simulink model "cddDcmApp"  (R2022b) (continued)
== system cddDcmApp/cddDcmApp_20ms_Runnable_sys == (continued)
  VgaIOCtrlService_FreezeMotorCurrentPosition : FunctionCaller  FunctionPrototype=ERR = VgaIOCtrlService_FreezeMotorCurrentPosition(Channel)  InputArgumentSpecifications=VGAChlType(0)  OutputArgumentSpecifications=P_Std_ReturnType
  VgaIOCtrlService_MotorReturnToECU : FunctionCaller  FunctionPrototype=ERR = VgaIOCtrlService_MotorReturnToECU(Channel)  InputArgumentSpecifications=VGAChlType(0)  OutputArgumentSpecifications=P_Std_ReturnType
  VgaIOCtrlService_MotorShortTermAdj : FunctionCaller  FunctionPrototype=ERR = VgaIOCtrlService_MotorShortTermAdj(Channel,TempValue)  InputArgumentSpecifications=VGAChlType(0), uint16(1)  OutputArgumentSpecifications=P_Std_ReturnType
  VgaParaService_GetAllMotorCaliStatus : FunctionCaller  FunctionPrototype=[Status,ERR] = VgaParaService_GetAllMotorCaliStatus()  OutputArgumentSpecifications=uint8(1), P_Std_ReturnType
  VgaParaService_GetCailRoutineStatus : FunctionCaller  FunctionPrototype=[Status,ERR] = VgaParaService_GetCailRoutineStatus()  OutputArgumentSpecifications=MtrRoutStaEnum(0), P_Std_ReturnType
  VgaParaService_GetFreshRecErrorStatus : FunctionCaller  FunctionPrototype=[Status,ERR] = VgaParaService_GetFreshRecErrorStatus()  OutputArgumentSpecifications=uint8(1), P_Std_ReturnType
  VgaParaService_GetFrntVgaErrorStatus : FunctionCaller  FunctionPrototype=[Status,ERR] = VgaParaService_GetFrntVgaErrorStatus()  OutputArgumentSpecifications=uint8(1), P_Std_ReturnType
  VgaParaService_GetMotorAllowSleepSts : FunctionCaller  FunctionPrototype=[Status,ERR] = VgaParaService_GetMotorAllowSleepSts()  OutputArgumentSpecifications=uint8(1), P_Std_ReturnType
  VgaParaService_GetMotorCaliStatus : FunctionCaller  FunctionPrototype=[Status,ERR] = VgaParaService_GetMotorCaliStatus(Channel)  InputArgumentSpecifications=VGAChlType(0)  OutputArgumentSpecifications=uint8(1), P_Std_ReturnType
  VgaParaService_GetMotorCurrentPosition : FunctionCaller  FunctionPrototype=[Pos,ERR] = VgaParaService_GetMotorCurrentPosition(Channel)  InputArgumentSpecifications=VGAChlType(0)  OutputArgumentSpecifications=uint8(1), P_Std_ReturnType
  VgaParaService_GetMotorCurrentPositionNew : FunctionCaller  FunctionPrototype=[Pos,ERR] = VgaParaService_GetMotorCurrentPositionNew(Channel)  InputArgumentSpecifications=VGAChlType(0)  OutputArgumentSpecifications=uint16(1), P_Std_ReturnType
  VgaParaService_GetMotorDidWorkingStatus : FunctionCaller  FunctionPrototype=[Status,ERR] = VgaParaService_GetMotorDidWorkingStatus(Channel)  InputArgumentSpecifications=VGAChlType(0)  OutputArgumentSpecifications=uint8(1), P_Std_ReturnType
  VgaParaService_GetMotorMoveCnt : FunctionCaller  FunctionPrototype=[Cnt,ERR] = VgaParaService_GetMotorMoveCnt(Channel)  InputArgumentSpecifications=VGAChlType(0)  OutputArgumentSpecifications=uint32(1), P_Std_ReturnType
  VgaParaService_GetMotorReqPosition : FunctionCaller  FunctionPrototype=[Position,ERR] = VgaParaService_GetMotorReqPosition(Channel)  InputArgumentSpecifications=VGAChlType(0)  OutputArgumentSpecifications=uint8(1), P_Std_ReturnType
  VgaParaService_GetMotorStepRange : FunctionCaller  FunctionPrototype=[Range,ERR] = VgaParaService_GetMotorStepRange(Channel)  InputArgumentSpecifications=VGAChlType(0)  OutputArgumentSpecifications=uint16(1), P_Std_ReturnType
  VgaParaService_GetMotorWorkingStatus : FunctionCaller  FunctionPrototype=[Status,ERR] = VgaParaService_GetMotorWorkingStatus(Channel)  InputArgumentSpecifications=VGAChlType(0)  OutputArgumentSpecifications=uint8(1), P_Std_ReturnType
  VgaParaService_GetRearVgaErrorStatus : FunctionCaller  FunctionPrototype=[Status,ERR] = VgaParaService_GetRearVgaErrorStatus()  OutputArgumentSpecifications=uint8(1), P_Std_ReturnType
  VgaParaService_SetCailRoutineStatus : FunctionCaller  FunctionPrototype=ERR = VgaParaService_SetCailRoutineStatus(Status)  InputArgumentSpecifications=MtrRoutStaEnum(0)  OutputArgumentSpecifications=P_Std_ReturnType
  VgaParaService_SetMotorMoveCnt : FunctionCaller  FunctionPrototype=ERR = VgaParaService_SetMotorMoveCnt(Channel,Cnt)  InputArgumentSpecifications=VGAChlType(0), uint32(1)  OutputArgumentSpecifications=P_Std_ReturnType
  VgaParaService_SetMotorRequestPosition : FunctionCaller  FunctionPrototype=ERR = VgaParaService_SetMotorRequestPosition(Channel,Present)  InputArgumentSpecifications=VGAChlType(0), uint16(1)  OutputArgumentSpecifications=P_Std_ReturnType
  VgaParaService_SetMotorStepRange : FunctionCaller  FunctionPrototype=ERR = VgaParaService_SetMotorStepRange(Channel,Range)  InputArgumentSpecifications=VGAChlType(0), uint16(1)  OutputArgumentSpecifications=P_Std_ReturnType
  wires:
  AACAirClngErrStsNgcc_AACAirClngErrStsNgcc_read/1 -> Terminator_1/1
  AACAirClngStsNgcc_AACAirClngStsNgcc_read/1 -> Terminator_2/1
  AfuFrameSts_AfuFrameSts_read/1 -> Terminator_3/1
  AFUGenErr_AFUGenErr_read/1 -> Terminator_4/1
  AirClngErrSts_AirClngErrSts_read/1 -> Terminator_5/1
  AirClngSts_AirClngSts_read/1 -> Terminator_6/1
  AirFragFanErr_AirFragFanErr_read/1 -> Terminator_7/1
  AirFragMot1Err_AirFragMot1Err_read/1 -> Terminator_8/1
  AirFragMot2Err_AirFragMot2Err_read/1 -> Terminator_9/1
  AirFragMot3Err_AirFragMot3Err_read/1 -> Terminator_10/1
  AirFragMot4Err_AirFragMot4Err_read/1 -> Terminator_11/1
  AirFragMot5Err_AirFragMot5Err_read/1 -> Terminator_12/1
  AirFragOvrVoltErr_AirFragOvrVoltErr_read/1 -> Terminator_13/1
  AirFragTempErr_AirFragTempErr_read/1 -> Terminator_14/1
  AirFragUndVoltErr_AirFragUndVoltErr_read/1 -> Terminator_15/1
  AirTFromHeatgEstimdFirstLeForCCM_AirTFromHeatgEstimdFirstLeForCCM_read/1 -> Terminator_16/1
  AirTFromHeatgEstimdFirstRiForCCM_AirTFromHeatgEstimdFirstRiForCCM_read/1 -> Terminator_17/1
  AirTFromHeatgEstimdForCCMForRead_AirTFromHeatgEstimdForCCMForRead_read/1 -> Terminator_18/1
  AirTFromHeatgEstimdReForCCM_AirTFromHeatgEstimdReForCCM_read/1 -> Terminator_19/1
  AirTFromHeatgEstimdSecLeForCCM_AirTFromHeatgEstimdSecLeForCCM_read/1 -> Terminator_20/1
  AiuFrameSts_AiuFrameSts_read/1 -> Terminator_21/1
  AmbTFildForClimaNgcc_AmbTFildForClima_read/1 -> Terminator_22/1
  AmbTRaw_AmbTRaw_read/1 -> Terminator_23/1
  AQSAirQuality_AQSAirQuality_read/1 -> Terminator_24/1
  AQSCOxstatus_AQSCOxstatus_read/1 -> Terminator_25/1
  AqsFrameSts_AqsFrameSts_read/1 -> Terminator_26/1
  AQSNH3status_AQSNH3status_read/1 -> Terminator_27/1
  AQSNoxStatus_AQSNoxStatus_read/1 -> Terminator_28/1
  BkpOfDstTrvld_BkpOfDstTrvld_read/1 -> Terminator_29/1
  BkpOfDstTrvld_BkpOfDstTrvld_IsUpdated_read/1 -> Terminator_30/1
  CarCfgForClimaCtrl_CarCfgForAirClng_read/1 -> Terminator_31/1
  CarCfgForClimaCtrl_CarCfgForAirClnrAdv_read/1 -> Terminator_32/1
  CarCfgForClimaCtrl_CarCfgForAirFrag_read/1 -> Terminator_33/1
  CarCfgForClimaCtrl_CarCfgForAirMtrlSnsr_read/1 -> Terminator_34/1
  CarCfgForClimaCtrl_CarCfgForAirQly_read/1 -> Terminator_35/1
  CarCfgForClimaCtrl_CarCfgForAmntOfSeat_read/1 -> Terminator_36/1
  CarCfgForClimaCtrl_CarCfgForAutDemst_read/1 -> Terminator_37/1
  CarCfgForClimaCtrl_CarCfgForAuxWtrPmp_read/1 -> Terminator_38/1
  CarCfgForClimaCtrl_CarCfgForBRAND_read/1 -> Terminator_39/1
  CarCfgForClimaCtrl_CarCfgForBypVlvInHvac_read/1 -> Terminator_40/1
  CarCfgForClimaCtrl_CarCfgForCabinOxgenSupply_read/1 -> Terminator_41/1
  CarCfgForClimaCtrl_CarCfgForClsVlvInHvac_read/1 -> Terminator_42/1
  CarCfgForClimaCtrl_CarCfgForCmprAcTyp_read/1 -> Terminator_43/1
  CarCfgForClimaCtrl_CarCfgForCmptmtClng_read/1 -> Terminator_44/1
  CarCfgForClimaCtrl_CarCfgForElectricLiftingModule_read/1 -> Terminator_45/1
  CarCfgForClimaCtrl_CarCfgForElecVentAdjmt_read/1 -> Terminator_46/1
  CarCfgForClimaCtrl_CarCfgForExtVentnClimaCtrl_read/1 -> Terminator_47/1
  CarCfgForClimaCtrl_CarCfgForFlcTyp_read/1 -> Terminator_48/1
  CarCfgForClimaCtrl_CarCfgForFloatingDriving_read/1 -> Terminator_49/1
  CarCfgForClimaCtrl_CarCfgForFoldCSD_read/1 -> Terminator_50/1
  CarCfgForClimaCtrl_CarCfgForFuTyp_read/1 -> Terminator_51/1
  CarCfgForClimaCtrl_CarCfgForGlassTyp_read/1 -> Terminator_52/1
  CarCfgForClimaCtrl_CarCfgForHeadRadar_read/1 -> Terminator_53/1
  CarCfgForClimaCtrl_CarCfgForHeatPmp_read/1 -> Terminator_54/1
  CarCfgForClimaCtrl_CarCfgForHeatrDurgDrvg_read/1 -> Terminator_55/1
  CarCfgForClimaCtrl_CarCfgForHeatrTyp_read/1 -> Terminator_56/1
  CarCfgForClimaCtrl_CarCfgForHvacFilTyp_read/1 -> Terminator_57/1
  CarCfgForClimaCtrl_CarCfgForHvacFlrSnsrForT_read/1 -> Terminator_58/1
  CarCfgForClimaCtrl_CarCfgForHvacHeatrSnsrT_read/1 -> Terminator_59/1
  CarCfgForClimaCtrl_CarCfgForHvacRe_read/1 -> Terminator_60/1
  CarCfgForClimaCtrl_CarCfgForHvacTyp_read/1 -> Terminator_61/1
  CarCfgForClimaCtrl_CarCfgForInteriorViewCamera_read/1 -> Terminator_62/1
  CarCfgForClimaCtrl_CarCfgForLifeDetection_read/1 -> Terminator_63/1
  CarCfgForClimaCtrl_CarCfgForLoVoltElecCfg_read/1 -> Terminator_64/1
  CarCfgForClimaCtrl_CarCfgForNavSysandIntnmap_read/1 -> Terminator_65/1
  CarCfgForClimaCtrl_CarCfgForNrOfDoor_read/1 -> Terminator_66/1
  CarCfgForClimaCtrl_CarCfgForPipeInFuTank_read/1 -> Terminator_67/1
  CarCfgForClimaCtrl_CarCfgForPowerSeats_read/1 -> Terminator_68/1
  CarCfgForClimaCtrl_CarCfgForPreClng_read/1 -> Terminator_69/1
  CarCfgForClimaCtrl_CarCfgForPrkgCDSNotAvl_read/1 -> Terminator_70/1
  CarCfgForClimaCtrl_CarCfgForPrkgClima_read/1 -> Terminator_71/1
  CarCfgForClimaCtrl_CarCfgForPrpsnTyp_read/1 -> Terminator_72/1
  CarCfgForClimaCtrl_CarCfgForPTCInFrontHVAC_read/1 -> Terminator_73/1
  CarCfgForClimaCtrl_CarCfgForRemEngStrtPreCdn_read/1 -> Terminator_74/1
  CarCfgForClimaCtrl_CarCfgForRoofHatch_read/1 -> Terminator_75/1
  CarCfgForClimaCtrl_CarCfgForSeatHeatgCal_read/1 -> Terminator_76/1
  CarCfgForClimaCtrl_CarCfgForSeatHeatgForRowFirst_read/1 -> Terminator_77/1
  CarCfgForClimaCtrl_CarCfgForSeatHeatgForRowSec_read/1 -> Terminator_78/1
  CarCfgForClimaCtrl_CarCfgForSeatVentnForRowFirst_read/1 -> Terminator_79/1
  CarCfgForClimaCtrl_CarCfgForSeatVentnForRowSec_read/1 -> Terminator_80/1
  CarCfgForClimaCtrl_CarCfgForSecRowVentnCenter_read/1 -> Terminator_81/1
  CarCfgForClimaCtrl_CarCfgForSecRowVentnSide_read/1 -> Terminator_82/1
  CarCfgForClimaCtrl_CarCfgForSmallFlowCyc_read/1 -> Terminator_83/1
  CarCfgForClimaCtrl_CarCfgForSmkSnsr_read/1 -> Terminator_84/1
  CarCfgForClimaCtrl_CarCfgForSnsrForCmptmtT_read/1 -> Terminator_85/1
  CarCfgForClimaCtrl_CarCfgForSnsrHumPrsnt_read/1 -> Terminator_86/1
  CarCfgForClimaCtrl_CarCfgForStartDevice_read/1 -> Terminator_87/1
  CarCfgForClimaCtrl_CarCfgForSteerWhlHeatg_read/1 -> Terminator_88/1
  CarCfgForClimaCtrl_CarCfgForSteerWhlPosn_read/1 -> Terminator_89/1
  CarCfgForClimaCtrl_CarCfgForSunSnsr_read/1 -> Terminator_90/1
  CarCfgForClimaCtrl_CarCfgForTelmNodTyp_read/1 -> Terminator_91/1
  CarCfgForClimaCtrl_CarCfgForTEMPEREDSTORAGEBOX_read/1 -> Terminator_92/1
  CarCfgForClimaCtrl_CarCfgForThermMngt_read/1 -> Terminator_93/1
  CarCfgForClimaCtrl_CarCfgForVehTyp_read/1 -> Terminator_94/1
  CarCfgForClimaCtrl_CarCfgForVentLiftMotorExiSts_read/1 -> Terminator_95/1
  CarCfgForClimaCtrl_CarCfgForVgaMotorExistSts_read/1 -> Terminator_96/1
  CarCfgForClimaCtrl_CarCfgForWadingAssistSystem_read/1 -> Terminator_97/1
  CarCfgForClimaCtrl_CarCfgForWindHeatd_read/1 -> Terminator_98/1
  CarCfgForClimaCtrl_CarCfgWindFollowHumanMov_read/1 -> Terminator_99/1
  CarTiGlb_CarTiGlb_read/1 -> Terminator_100/1
  CarTiGlb_CarTiGlb_IsUpdated_read/1 -> Terminator_101/1
  CcmIntCo2Lvl_CcmIntCo2Lvl_read/1 -> Terminator_102/1
  CCMLIN1frameSts_CCMLIN1frameSts_read/1 -> Terminator_103/1
  CCMLIN2frameSts_CCMLIN2frameSts_read/1 -> Terminator_104/1
  CCMLIN3frameSts_CCMLIN3frameSts_read/1 -> Terminator_105/1
  CdsFrameSts_CdsFrameSts_read/1 -> Terminator_106/1
  ClimaObsActv_ClimaObsActv_read/1 -> Terminator_107/1
  CmpmtInAirPmAQI_CmpmtInAirPmAQI_read/1 -> Terminator_108/1
  CmpmtInAirPmDnsty_CmpmtInAirPmDnsty_read/1 -> Terminator_109/1
  CmptmtAirTEstimdAtRowFirst_CmptmtAirTEstimdAtRowFirst_read/1 -> Terminator_110/1
  CmptmtAirTEstimdAtRowFirstIni_CmptmtAirTEstimdAtRowFirstIni_read/1 -> Terminator_111/1
  CmptmtAirTEstimdAtRowSec_CmptmtAirTEstimdAtRowSec_read/1 -> Terminator_112/1
  CmptmtAirTEstimdAtRowSecIni_CmptmtAirTEstimdAtRowSecIni_read/1 -> Terminator_113/1
  CmptmtAirTEstimdFirstNvRamIni_CmptmtAirTEstimdFirstNvRamIni_read/1 -> Terminator_114/1
  CmptmtAirTEstimdSecNvRamIni_CmptmtAirTEstimdSecNvRamIni_read/1 -> Terminator_115/1
  CmptmtFrntWindDewT_CmptmtFrntWindDewT_read/1 -> Terminator_116/1
  CmptmtFrntWindT_CmptmtFrntWindT_read/1 -> Terminator_117/1
  CmptmtOxygenConcVluNgcc_CmptmtOxygenConcVluNgcc_read/1 -> Terminator_118/1
  CmptmtRelHum_CmptmtRelHum_read/1 -> Terminator_119/1
  CmptmtRelHum_CmptmtRelHum_IsUpdated_read/1 -> Terminator_120/1
  CmptmtTRearRaw_CmptmtTRearRaw_read/1 -> Terminator_121/1
  CoupldCtrlErrAtRowFirstLe_CoupldCtrlErrAtRowFirstLe_read/1 -> Terminator_122/1
  CoupldCtrlErrAtRowFirstRi_CoupldCtrlErrAtRowFirstRi_read/1 -> Terminator_123/1
  CoupldCtrlErrAtRowSecLe_CoupldCtrlErrAtRowSecLe_read/1 -> Terminator_124/1
  CoupldCtrlErrAtRowSecRi_CoupldCtrlErrAtRowSecRi_read/1 -> Terminator_125/1
  DcMotorCurPosVol_DcMotorCurPosVol_read/1 -> Terminator_126/1
  EngT_Ngcc_EngT_read/1 -> Terminator_127/1
  EvaprTFrntFildForClima_EvaprTFrntFildForClima_read/1 -> Terminator_128/1
  EvaprTReFildForClima_EvaprTReFildForClima_read/1 -> Terminator_129/1
  HmiHvacFanLvlFrnt_HmiHvacFanLvlFrnt_read/1 -> Terminator_130/1
  HmiHvacFanLvlRe_HmiHvacFanLvlRe_read/1 -> Terminator_131/1
  HvacAirTEstimdAtRowFirstLe_HvacAirTEstimdAtRowFirstLe_read/1 -> Terminator_132/1
  HvacAirTEstimdAtRowFirstRi_HvacAirTEstimdAtRowFirstRi_read/1 -> Terminator_133/1
  HvacAirTEstimdAtRowSecLe_HvacAirTEstimdAtRowSecLe_read/1 -> Terminator_134/1
  HvacAirTEstimdAtRowSecRi_HvacAirTEstimdAtRowSecRi_read/1 -> Terminator_135/1
  HvacAirTReqAtRowFirstLe_HvacAirTReqAtRowFirstLe_read/1 -> Terminator_136/1
  HvacAirTReqAtRowFirstRi_HvacAirTReqAtRowFirstRi_read/1 -> Terminator_137/1
  HvacAirTReqAtRowSecLe_HvacAirTReqAtRowSecLe_read/1 -> Terminator_138/1
  HvacAirTReqAtRowSecRi_HvacAirTReqAtRowSecRi_read/1 -> Terminator_139/1
  HvacCfgForClimaCtrl_HvacCfgForAQSTypes_read/1 -> Terminator_140/1
  HvacCfgForClimaCtrl_HvacCfgForHvacFrontBlowerType_read/1 -> Terminator_141/1
  HvacCfgForClimaCtrl_HvacCfgForHvacLowSideRETXVESts_read/1 -> Terminator_142/1
  HvacCfgForClimaCtrl_HvacCfgForHvacMiscExistSts_read/1 -> Terminator_143/1
  HvacCfgForClimaCtrl_HvacCfgForHvacMotorExistSts_read/1 -> Terminator_144/1
  HvacCfgForClimaCtrl_HvacCfgForHvacReBlowerType_read/1 -> Terminator_145/1
  HvacCfgForClimaCtrl_HvacCfgForHvacReVentPstn_read/1 -> Terminator_146/1
  HvacCfgForClimaCtrl_HvacCfgForHvacSensorExistSts_read/1 -> Terminator_147/1
  HvacCfgForClimaCtrl_HvacCfgForHvacSnsrRCmptmtESts_read/1 -> Terminator_148/1
  HvacCfgForClimaCtrl_HvacCfgForHvacSnsrReHexESts_read/1 -> Terminator_149/1
  HvacFanSet3HvacFanSpd1_HvacFanSet3HvacFanSpd1_read/1 -> Terminator_150/1
  HvacFanSts2HvacFanBattI2_HvacFanSts2HvacFanBattI2_read/1 -> Terminator_151/1
  HvacFanSts2HvacFanBattU2_HvacFanSts2HvacFanBattU2_read/1 -> Terminator_152/1
  HvacFanSts2HvacFanMotU2_HvacFanSts2HvacFanMotU2_read/1 -> Terminator_153/1
  HvacFanSts2HvacFanReSts1_HvacFanSts2HvacFanReSts1_read/1 -> Terminator_154/1
  HvacFanSts2HvacFanReSts2_HvacFanSts2HvacFanReSts2_read/1 -> Terminator_155/1
  HvacFanSts3HvacFanBattI_HvacFanSts3HvacFanBattI_read/1 -> Terminator_156/1
  HvacFanSts3HvacFanBattU_HvacFanSts3HvacFanBattU_read/1 -> Terminator_157/1
  HvacFanSts3HvacFanCircShoOrMotBlkd1_HvacFanSts3HvacFanCircShoOrMotBlkd1_read/1 -> Terminator_158/1
  HvacFanSts3HvacFanDiagFlag1_HvacFanSts3HvacFanDiagFlag1_read/1 -> Terminator_159/1
  HvacFanSts3HvacFanILimn_HvacFanSts3HvacFanILimn_read/1 -> Terminator_160/1
  HvacFanSts3HvacFanIntErr1_HvacFanSts3HvacFanIntErr1_read/1 -> Terminator_161/1
  HvacFanSts3HvacFanSpdFd_HvacFanSts3HvacFanSpdFd_read/1 -> Terminator_162/1
  HvacFanSts3HvacFanTErr_HvacFanSts3HvacFanTErr_read/1 -> Terminator_163/1
  HvacFanSts3HvDetd_HvacFanSts3HvDetd_read/1 -> Terminator_164/1
  HvacFanSts3OverCurrErr_HvacFanSts3OverCurrErr_read/1 -> Terminator_165/1
  HvacFanSts3UDetdLo_HvacFanSts3UDetdLo_read/1 -> Terminator_166/1
  HvacFanVoltReqFrnt_HvacFanVoltReqFrnt_read/1 -> Terminator_167/1
  HvacFanVoltReqRe_HvacFanVoltReqRe_read/1 -> Terminator_168/1
  HvacLpmUFbRe_HvacLpmUFbRe_read/1 -> Terminator_169/1
  HvacPwm2MosVFbFrnt_HvacPwm2MosVFbFrnt_read/1 -> Terminator_170/1
  HvacPwmSignErr_HvacPwmSignErr_read/1 -> Terminator_171/1
  InPm25FanErr_InPm25FanErr_read/1 -> Terminator_172/1
  InPm25SensrIntErr_InPm25SensrIntErr_read/1 -> Terminator_173/1
  InPm25SnrTempErr_InPm25SnrTempErr_read/1 -> Terminator_174/1
  InPm25SnrVoltgRangErr_InPm25SnrVoltgRangErr_read/1 -> Terminator_175/1
  InPm25SnsrElecErr_InPm25SnsrElecErr_read/1 -> Terminator_176/1
  IntCo2Conc_IntCo2Conc_read/1 -> Terminator_177/1
  IntCo2Lvl_IntCo2Lvl_read/1 -> Terminator_178/1
  LdAdVal_CurrAdVal_read/1 -> Terminator_179/1
  LdAdVal_ValidAdVal_read/1 -> Terminator_180/1
  MemReadForCenVentLiftModSts_MemReadForCenVentLiftModSts_read/1 -> Terminator_181/1
  MemReadForClimaEgyMgrForDID_MemReadForClimaEgyMgrForDID_read/1 -> Terminator_182/1
  NvMBlockCCMLoadDataArray_NvMBlockCCMLoadDataArray_read/1 -> Terminator_183/1
  NvMBlockDcMtrCalDataArray_NvMBlockDcMtrCalDataArray_read/1 -> Terminator_184/1
  ObsvrAirTOffsFromWindTIni_ObsvrAirTOffsFromWindTIni_read/1 -> Terminator_185/1
  ObsvrCmptmtTIninActv_ObsvrCmptmtTIninActv_read/1 -> Terminator_186/1
  ObsvrCmptmtTRamRxInt_ObsvrCmptmtTRamRxInt_read/1 -> Terminator_187/1
  ObsvrInCarTFrntIni_ObsvrInCarTFrntIni_read/1 -> Terminator_188/1
  ObsvrInCarTRearIni_ObsvrInCarTRearIni_read/1 -> Terminator_189/1
  ObsvrIntrMtrlTIni_ObsvrIntrMtrlTIni_read/1 -> Terminator_190/1
  OGMIntErrNgcc_OGMIntErrNgcc_read/1 -> Terminator_191/1
  OGMOvrVoltRangErrNgcc_OGMOvrVoltRangErrNgcc_read/1 -> Terminator_192/1
  OGMTempErrNgcc_OGMTempErrNgcc_read/1 -> Terminator_193/1
  OGMUndVoltRangErrNgcc_OGMUndVoltRangErrNgcc_read/1 -> Terminator_194/1
  OutdAirQly_OutdAirQly_read/1 -> Terminator_195/1
  OxygenConcSnsrElecErrNgcc_OxygenConcSnsrElecErrNgcc_read/1 -> Terminator_196/1
  OxygenConcSnsrIntErrNgcc_OxygenConcSnsrIntErrNgcc_read/1 -> Terminator_197/1
  OxygenConcSnsrOvrVoltRangErrNgcc_OxygenConcSnsrOvrVoltRangErrNgcc_read/1 -> Terminator_198/1
  OxygenConcSnsrTempErrNgcc_OxygenConcSnsrTempErrNgcc_read/1 -> Terminator_199/1
  OxygenConcSnsrUndVoltRangErrNgcc_OxygenConcSnsrUndVoltRangErrNgcc_read/1 -> Terminator_200/1
  PmsiFrameSts_PmsiFrameSts_read/1 -> Terminator_201/1
  ReEvapVlvSts_ReEvapVlvSts_read/1 -> Terminator_202/1
  ReHvacActrElecErr_ReHvacActrElecErr_read/1 -> Terminator_203/1
  ReHvacActrOvrTempErr_ReHvacActrOvrTempErr_read/1 -> Terminator_204/1
  ReHvacActrVoltErr_ReHvacActrVoltErr_read/1 -> Terminator_205/1
  RelHumSnsrRelHum_RelHumSnsrRelHum_read/1 -> Terminator_206/1
  RhfaFrameSts_RhfaFrameSts_read/1 -> Terminator_207/1
  SensorAdValue_SensorAdValue_read/1 -> Terminator_208/1
  SrsAdVal_CurrAdVal_read/1 -> Terminator_209/1
  SrsAdVal_ValidAdVal_read/1 -> Terminator_210/1
  SunData_SunData_read/1 -> Terminator_211/1
  SWVersion_SWVersion_read/1 -> Terminator_212/1
  SysServCarCfgSts_SysServCarID_read/1 -> Terminator_213/1
  SysServCarCfgSts_SysServCCPAQSSts_read/1 -> Terminator_214/1
  SysServCarCfgSts_SysServCCPBGMNum_read/1 -> Terminator_215/1
  SysServCarCfgSts_SysServCCPHEXSensorSts_read/1 -> Terminator_216/1
  SysServCarCfgSts_SysServCCPLin2AFUSts_read/1 -> Terminator_217/1
  SysServCarCfgSts_SysServCCPLin2AIUSts_read/1 -> Terminator_218/1
  SysServCarCfgSts_SysServCCPLin2AQSSts_read/1 -> Terminator_219/1
  SysServCarCfgSts_SysServCCPLin2CDSSts_read/1 -> Terminator_220/1
  SysServCarCfgSts_SysServCCPLin2PMSISts_read/1 -> Terminator_221/1
  SysServCarCfgSts_SysServCCPLin3VGASts_read/1 -> Terminator_222/1
  SysServCarCfgSts_SysServDUAirVentSnrSts_read/1 -> Terminator_223/1
  SysServCarCfgSts_SysServDUBlowerPwmSignSts_read/1 -> Terminator_224/1
  SysServCarCfgSts_SysServDUBlowerPwmSignTypeSts_read/1 -> Terminator_225/1
  SysServCarCfgSts_SysServDUBlowerPwmSts_read/1 -> Terminator_226/1
  SysServCarCfgSts_SysServDUDCDefrostSts_read/1 -> Terminator_227/1
  SysServCarCfgSts_SysServDUDCFLMdeSts_read/1 -> Terminator_228/1
  SysServCarCfgSts_SysServDUDCModeSts_read/1 -> Terminator_229/1
  SysServCarCfgSts_SysServDUDCRecSts_read/1 -> Terminator_230/1
  SysServCarCfgSts_SysServDUDCSModeSts_read/1 -> Terminator_231/1
  SysServCarCfgSts_SysServDUDCSTLSts_read/1 -> Terminator_232/1
  SysServCarCfgSts_SysServDUDCTLSts_read/1 -> Terminator_233/1
  SysServCarCfgSts_SysServDUDCTRSts_read/1 -> Terminator_234/1
  SysServCarCfgSts_SysServDUFLFootSts_read/1 -> Terminator_235/1
  SysServCarCfgSts_SysServDUFLVentSts_read/1 -> Terminator_236/1
  SysServCarCfgSts_SysServDUFRFootSts_read/1 -> Terminator_237/1
  SysServCarCfgSts_SysServDUFRVentSts_read/1 -> Terminator_238/1
  SysServCarCfgSts_SysServDUIncarMotorSts_read/1 -> Terminator_239/1
  SysServCarCfgSts_SysServDULin1HBMFSts_read/1 -> Terminator_240/1
  SysServCarCfgSts_SysServDULin4HFAFSts_read/1 -> Terminator_241/1
  SysServCarCfgSts_SysServDULin4HFAFStsH_read/1 -> Terminator_242/1
  SysServCarCfgSts_SysServDUReBlowerPwmSignSts_read/1 -> Terminator_243/1
  SysServCarCfgSts_SysServDUReBlowerPwmSts_read/1 -> Terminator_244/1
  SysServCarCfgSts_SysServDUSFLFootSts_read/1 -> Terminator_245/1
  SysServCarCfgSts_SysServDUSFLVentSts_read/1 -> Terminator_246/1
  SysServCarCfgSts_SysServDUSFRFootSts_read/1 -> Terminator_247/1
  SysServCarCfgSts_SysServDUSFRVentSts_read/1 -> Terminator_248/1
  SysServCarCfgSts_SysServDUSnsrForFCmptmtTSts_read/1 -> Terminator_249/1
  SysServCarCfgSts_SysServDUSnsrForRair_read/1 -> Terminator_250/1
  SysServCarCfgSts_SysServDUSnsrForRCmptmtTSts_read/1 -> Terminator_251/1
  SysServCarCfgSts_SysServFrntHEXSnsrPosn_read/1 -> Terminator_252/1
  SysServCarCfgSts_SysServLowSideRETXVSts_read/1 -> Terminator_253/1
  SysServCarCfgSts_SysServReHEXSnsrPosn_read/1 -> Terminator_254/1
  SysServCarCfgSts_SysServRHFASts_read/1 -> Terminator_255/1
  SysServCarCfgSts_SysServSnsrForFCmptmtTType_read/1 -> Terminator_256/1
  TestRunCriteriaTRC_TestRunCriteriaTRC_read/1 -> Terminator_257/1
  TiOfDeactvnForClimaObs_TiOfDeactvnForClimaObs_read/1 -> Terminator_258/1
  VbmrFrameSts_VbmrFrameSts_read/1 -> Terminator_259/1
  VehBattU_VehBattU_read/1 -> Terminator_260/1
  VehBattU_VehBattU_IsUpdated_read/1 -> Terminator_261/1
  VehModMngtGlbSafe1_VehModMngtGlbSafe1_read/1 -> Terminator_262/1
  VehModMngtGlbSafe1_VehModMngtGlbSafe1_IsUpdated_read/1 -> Terminator_263/1
  VentnActr01ElecErr_VentnActr01ElecErr_read/1 -> Terminator_264/1
  VentnActr01OvrTempErr_VentnActr01OvrTempErr_read/1 -> Terminator_265/1
  VentnActr01VoltErr_VentnActr01VoltErr_read/1 -> Terminator_266/1
  VentnActr02ElecErr_VentnActr02ElecErr_read/1 -> Terminator_267/1
  VentnActr02OvrTempErr_VentnActr02OvrTempErr_read/1 -> Terminator_268/1
  VentnActr02VoltErr_VentnActr02VoltErr_read/1 -> Terminator_269/1
  VentnActr03ElecErr_VentnActr03ElecErr_read/1 -> Terminator_270/1
  VentnActr03OvrTempErr_VentnActr03OvrTempErr_read/1 -> Terminator_271/1
  VentnActr03VoltErr_VentnActr03VoltErr_read/1 -> Terminator_272/1
  VentnActr04ElecErr_VentnActr04ElecErr_read/1 -> Terminator_273/1
  VentnActr04OvrTempErr_VentnActr04OvrTempErr_read/1 -> Terminator_274/1
  VentnActr04VoltErr_VentnActr04VoltErr_read/1 -> Terminator_275/1
  VentnActr05ElecErr_VentnActr05ElecErr_read/1 -> Terminator_276/1
  VentnActr05OvrTempErr_VentnActr05OvrTempErr_read/1 -> Terminator_277/1
  VentnActr05VoltErr_VentnActr05VoltErr_read/1 -> Terminator_278/1
  VentnActr06ElecErr_VentnActr06ElecErr_read/1 -> Terminator_279/1
  VentnActr06OvrTempErr_VentnActr06OvrTempErr_read/1 -> Terminator_280/1
  VentnActr06VoltErr_VentnActr06VoltErr_read/1 -> Terminator_281/1
  VentnActr07ElecErr_VentnActr07ElecErr_read/1 -> Terminator_282/1
  VentnActr07OvrTempErr_VentnActr07OvrTempErr_read/1 -> Terminator_283/1
  VentnActr07VoltErr_VentnActr07VoltErr_read/1 -> Terminator_284/1
  VentnActr08ElecErr_VentnActr08ElecErr_read/1 -> Terminator_285/1
  VentnActr08OvrTempErr_VentnActr08OvrTempErr_read/1 -> Terminator_286/1
  VentnActr08VoltErr_VentnActr08VoltErr_read/1 -> Terminator_287/1
  VentnActr09ElecErr_VentnActr09ElecErr_read/1 -> Terminator_288/1
  VentnActr09OvrTempErr_VentnActr09OvrTempErr_read/1 -> Terminator_289/1
  VentnActr09VoltErr_VentnActr09VoltErr_read/1 -> Terminator_290/1
  BswLifeCycle_LifeCycle_read/1 -> Terminator_291/1
  BatParaService_GetBatteryVolValue/1 -> Terminator_292/1
  BatParaService_GetBatteryVolValue/2 -> Terminator_293/1
  Ground_1/1 -> DcMotorIOCtrlService_FreezeMotorCurrentPosition/1
  DcMotorIOCtrlService_FreezeMotorCurrentPosition/1 -> Terminator_294/1
  Ground_2/1 -> DcMotorIOCtrlService_MotorRetCtlToECU/1
  DcMotorIOCtrlService_MotorRetCtlToECU/1 -> Terminator_295/1
  Ground_3/1 -> DcMotorIOCtrlService_MotorShortTermAdj/1
  Ground_4/1 -> DcMotorIOCtrlService_MotorShortTermAdj/2
  DcMotorIOCtrlService_MotorShortTermAdj/1 -> Terminator_296/1
  DcMotorParaService_DcMotorAllowSleepSts/1 -> Terminator_297/1
  DcMotorParaService_DcMotorAllowSleepSts/2 -> Terminator_298/1
  Ground_5/1 -> DcMotorParaService_GetMotorActualPositon/1
  DcMotorParaService_GetMotorActualPositon/1 -> Terminator_299/1
  DcMotorParaService_GetMotorActualPositon/2 -> Terminator_300/1
  DcMotorParaService_GetMotorCailLearnSts/1 -> Terminator_301/1
  DcMotorParaService_GetMotorCailLearnSts/2 -> Terminator_302/1
  Ground_6/1 -> DcMotorParaService_GetMotorCalibrationStatus/1
  DcMotorParaService_GetMotorCalibrationStatus/1 -> Terminator_303/1
  DcMotorParaService_GetMotorCalibrationStatus/2 -> Terminator_304/1
  Ground_7/1 -> DcMotorParaService_GetMotorDidWorkingStatus/1
  DcMotorParaService_GetMotorDidWorkingStatus/1 -> Terminator_305/1
  DcMotorParaService_GetMotorDidWorkingStatus/2 -> Terminator_306/1
  Ground_8/1 -> DcMotorParaService_GetMotorEndPosition/1
  DcMotorParaService_GetMotorEndPosition/1 -> Terminator_307/1
  DcMotorParaService_GetMotorEndPosition/2 -> Terminator_308/1
  Ground_9/1 -> DcMotorParaService_GetMotorRequestPercent/1
  DcMotorParaService_GetMotorRequestPercent/1 -> Terminator_309/1
  DcMotorParaService_GetMotorRequestPercent/2 -> Terminator_310/1
  DcMotorParaService_GetMotorRoutineCailSts/1 -> Terminator_311/1
  DcMotorParaService_GetMotorRoutineCailSts/2 -> Terminator_312/1
  Ground_10/1 -> DcMotorParaService_GetMotorRunTime/1
  DcMotorParaService_GetMotorRunTime/1 -> Terminator_313/1
  DcMotorParaService_GetMotorRunTime/2 -> Terminator_314/1
  Ground_11/1 -> DcMotorParaService_GetMotorWorkingStatus/1
  DcMotorParaService_GetMotorWorkingStatus/1 -> Terminator_315/1
  DcMotorParaService_GetMotorWorkingStatus/2 -> Terminator_316/1
  Ground_12/1 -> DcMotorParaService_GetMotorZeroPosition/1
  DcMotorParaService_GetMotorZeroPosition/1 -> Terminator_317/1
  DcMotorParaService_GetMotorZeroPosition/2 -> Terminator_318/1
  DcMotorParaService_GetRamAirErrorStatus/1 -> Terminator_319/1
  DcMotorParaService_GetRamAirErrorStatus/2 -> Terminator_320/1
  Ground_13/1 -> DcMotorParaService_SetMotorEndPosition/1
  Ground_14/1 -> DcMotorParaService_SetMotorEndPosition/2
  DcMotorParaService_SetMotorEndPosition/1 -> Terminator_321/1
  Ground_15/1 -> DcMotorParaService_SetMotorRequestPercent/1
  Ground_16/1 -> DcMotorParaService_SetMotorRequestPercent/2
  DcMotorParaService_SetMotorRequestPercent/1 -> Terminator_322/1
  DcMotorParaService_SetMotorRoutineCail/1 -> Terminator_323/1
  Ground_17/1 -> DcMotorParaService_SetMotorRoutineCailSts/1
  DcMotorParaService_SetMotorRoutineCailSts/1 -> Terminator_324/1
  Ground_18/1 -> DcMotorParaService_SetMotorRunTime/1
  Ground_19/1 -> DcMotorParaService_SetMotorRunTime/2
  DcMotorParaService_SetMotorRunTime/1 -> Terminator_325/1
  Ground_20/1 -> DcMotorParaService_SetMotorZeroPosition/1
  Ground_21/1 -> DcMotorParaService_SetMotorZeroPosition/2
  DcMotorParaService_SetMotorZeroPosition/1 -> Terminator_326/1
  HbmfParaService_FrntBlwrspdLIN1_FreezeCurrentState/1 -> Terminator_327/1
  HbmfParaService_FrntBlwrspdLIN1_ReturnControlToECU/1 -> Terminator_328/1
  Ground_22/1 -> HbmfParaService_FrntBlwrspdLIN1_ShortTermAdjustment/1
  HbmfParaService_FrntBlwrspdLIN1_ShortTermAdjustment/1 -> Terminator_329/1
  Ground_23/1 -> HbmfParaService_GetHbmfDvAsmPartNr/1
  HbmfParaService_GetHbmfDvAsmPartNr/1 -> Terminator_330/1
  HbmfParaService_GetHbmfDvAsmPartNr/2 -> Terminator_331/1
  HbmfParaService_GetHbmfPrvEcuSerNr/1 -> Terminator_332/1
  HbmfParaService_GetHbmfPrvEcuSerNr/2 -> Terminator_333/1
  HfaCaliService_SetAllCalibrate/1 -> Terminator_334/1
  HfaCaliService_SetAllParaInit/1 -> Terminator_335/1
  Ground_24/1 -> HfaCaliService_SetMotorCalibrate/1
  HfaCaliService_SetMotorCalibrate/1 -> Terminator_336/1
  Ground_25/1 -> HfaCaliService_SetMotorParaInit/1
  HfaCaliService_SetMotorParaInit/1 -> Terminator_337/1
  Ground_26/1 -> HfaIOCtrlService_FreezeMotorCurrentPosition/1
  HfaIOCtrlService_FreezeMotorCurrentPosition/1 -> Terminator_338/1
  Ground_27/1 -> HfaIOCtrlService_MotorReturnToECU/1
  HfaIOCtrlService_MotorReturnToECU/1 -> Terminator_339/1
  Ground_28/1 -> HfaIOCtrlService_MotorShortTermAdj/1
  Ground_29/1 -> HfaIOCtrlService_MotorShortTermAdj/2
  HfaIOCtrlService_MotorShortTermAdj/1 -> Terminator_340/1
  HfaParaService_GetAllMotorCaliStatus/1 -> Terminator_341/1
  HfaParaService_GetAllMotorCaliStatus/2 -> Terminator_342/1
  HfaParaService_GetCailRoutineStatus/1 -> Terminator_343/1
  HfaParaService_GetCailRoutineStatus/2 -> Terminator_344/1
  HfaParaService_GetFreshRecErrorStatus/1 -> Terminator_345/1
  HfaParaService_GetFreshRecErrorStatus/2 -> Terminator_346/1
  HfaParaService_GetMotorAllowSleepSts/1 -> Terminator_347/1
  HfaParaService_GetMotorAllowSleepSts/2 -> Terminator_348/1
  Ground_30/1 -> HfaParaService_GetMotorCaliStatus/1
  HfaParaService_GetMotorCaliStatus/1 -> Terminator_349/1
  HfaParaService_GetMotorCaliStatus/2 -> Terminator_350/1
  Ground_31/1 -> HfaParaService_GetMotorCurrentPosition/1
  HfaParaService_GetMotorCurrentPosition/1 -> Terminator_351/1
  HfaParaService_GetMotorCurrentPosition/2 -> Terminator_352/1
  Ground_32/1 -> HfaParaService_GetMotorDidWorkingStatus/1
  HfaParaService_GetMotorDidWorkingStatus/1 -> Terminator_353/1
  HfaParaService_GetMotorDidWorkingStatus/2 -> Terminator_354/1
  Ground_33/1 -> HfaParaService_GetMotorMoveCnt/1
  HfaParaService_GetMotorMoveCnt/1 -> Terminator_355/1
  HfaParaService_GetMotorMoveCnt/2 -> Terminator_356/1
  Ground_34/1 -> HfaParaService_GetMotorReqPosition/1
  HfaParaService_GetMotorReqPosition/1 -> Terminator_357/1
  HfaParaService_GetMotorReqPosition/2 -> Terminator_358/1
  Ground_35/1 -> HfaParaService_GetMotorStepRange/1
  HfaParaService_GetMotorStepRange/1 -> Terminator_359/1
  HfaParaService_GetMotorStepRange/2 -> Terminator_360/1
  Ground_36/1 -> HfaParaService_GetMotorWorkingStatus/1
  HfaParaService_GetMotorWorkingStatus/1 -> Terminator_361/1
  HfaParaService_GetMotorWorkingStatus/2 -> Terminator_362/1
  HfaParaService_GetRamAirErrorStatus/1 -> Terminator_363/1
  HfaParaService_GetRamAirErrorStatus/2 -> Terminator_364/1
  Ground_37/1 -> HfaParaService_SetCailRoutineStatus/1
  HfaParaService_SetCailRoutineStatus/1 -> Terminator_365/1
  Ground_38/1 -> HfaParaService_SetMotorMoveCnt/1
  Ground_39/1 -> HfaParaService_SetMotorMoveCnt/2
  HfaParaService_SetMotorMoveCnt/1 -> Terminator_366/1
  Ground_40/1 -> HfaParaService_SetMotorRequestPosition/1
  Ground_41/1 -> HfaParaService_SetMotorRequestPosition/2
  HfaParaService_SetMotorRequestPosition/1 -> Terminator_367/1
  Ground_42/1 -> HfaParaService_SetMotorStepRange/1
  Ground_43/1 -> HfaParaService_SetMotorStepRange/2
  HfaParaService_SetMotorStepRange/1 -> Terminator_368/1
  HfaRIDService_GetAutoAddreRoutineSts/1 -> Terminator_369/1
  HfaRIDService_GetAutoAddreRoutineSts/2 -> Terminator_370/1
  HfaRIDService_GetMotorAutoAddreSts/1 -> Terminator_371/1
  HfaRIDService_GetMotorAutoAddreSts/2 -> Terminator_372/1
  Ground_44/1 -> HfaRIDService_SetAutoAddreRoutineSts/1
  HfaRIDService_SetAutoAddreRoutineSts/1 -> Terminator_373/1
  HfaRIDService_SetMotorAutoAddre/1 -> Terminator_374/1
  Ground_45/1 -> IcuServ_GetDutyCycleValues/1
  IcuServ_GetDutyCycleValues/1 -> Terminator_375/1
  IcuServ_GetDutyCycleValues/2 -> Terminator_376/1
  IcuServ_IcuStart/1 -> Terminator_377/1
  Ground_46/1 -> IcuServ_SetMaxPwmPeriod/1
  Ground_47/1 -> IcuServ_SetMaxPwmPeriod/2
  IcuServ_SetMaxPwmPeriod/1 -> Terminator_378/1
  Ground_48/1 -> Lin2MgrParaServ_AFU_DvAsmPartNr/1
  Lin2MgrParaServ_AFU_DvAsmPartNr/1 -> Terminator_379/1
  Lin2MgrParaServ_AFU_DvAsmPartNr/2 -> Terminator_380/1
  Lin2MgrParaServ_AFU_DvAsmPartNr/3 -> Terminator_381/1
  Lin2MgrParaServ_AFU_PrvEcuSerNr/1 -> Terminator_382/1
  Lin2MgrParaServ_AFU_PrvEcuSerNr/2 -> Terminator_383/1
  Lin2MgrParaServ_AFU_PrvEcuSerNr/3 -> Terminator_384/1
  Ground_49/1 -> Lin2MgrParaServ_AIU_DvAsmPartNr/1
  Lin2MgrParaServ_AIU_DvAsmPartNr/1 -> Terminator_385/1
  Lin2MgrParaServ_AIU_DvAsmPartNr/2 -> Terminator_386/1
  Lin2MgrParaServ_AIU_DvAsmPartNr/3 -> Terminator_387/1
  Lin2MgrParaServ_AIU_PrvEcuSerNr/1 -> Terminator_388/1
  Lin2MgrParaServ_AIU_PrvEcuSerNr/2 -> Terminator_389/1
  Lin2MgrParaServ_AIU_PrvEcuSerNr/3 -> Terminator_390/1
  Ground_50/1 -> Lin2MgrParaServ_CDS_DvAsmPartNr/1
  Lin2MgrParaServ_CDS_DvAsmPartNr/1 -> Terminator_391/1
  Lin2MgrParaServ_CDS_DvAsmPartNr/2 -> Terminator_392/1
  Lin2MgrParaServ_CDS_DvAsmPartNr/3 -> Terminator_393/1
  Lin2MgrParaServ_CDS_PrvEcuSerNr/1 -> Terminator_394/1
  Lin2MgrParaServ_CDS_PrvEcuSerNr/2 -> Terminator_395/1
  Lin2MgrParaServ_CDS_PrvEcuSerNr/3 -> Terminator_396/1
  Ground_51/1 -> Lin2MgrParaServ_PMSI_DvAsmPartNr/1
  Lin2MgrParaServ_PMSI_DvAsmPartNr/1 -> Terminator_397/1
  Lin2MgrParaServ_PMSI_DvAsmPartNr/2 -> Terminator_398/1
  Lin2MgrParaServ_PMSI_DvAsmPartNr/3 -> Terminator_399/1
  Lin2MgrParaServ_PMSI_PrvEcuSerNr/1 -> Terminator_400/1
  Lin2MgrParaServ_PMSI_PrvEcuSerNr/2 -> Terminator_401/1
  Lin2MgrParaServ_PMSI_PrvEcuSerNr/3 -> Terminator_402/1
  Lin2ParaService_FreezeVbmrReqPercent/1 -> Terminator_403/1
  Lin2ParaService_ReadVbmrPercent/1 -> Terminator_404/1
  Lin2ParaService_ReadVbmrPercent/2 -> Terminator_405/1
  Lin2ParaService_VbmrReqPercentRetCtrltoECU/1 -> Terminator_406/1
  Ground_52/1 -> Lin2ParaService_VbmrReqPercentShortTermAdj/1
  Lin2ParaService_VbmrReqPercentShortTermAdj/1 -> Terminator_407/1
  LoadParaService_FreezeIncarReqStatus/1 -> Terminator_408/1
  LoadParaService_FreezeReTXVReqStatus/1 -> Terminator_409/1
  LoadParaService_FreezeVlclPwmPercent/1 -> Terminator_410/1
  LoadParaService_FreezeVlclRearPwmPercent/1 -> Terminator_411/1
  LoadParaService_IncarReqRetCtrltoECU/1 -> Terminator_412/1
  Ground_53/1 -> LoadParaService_IncarReqShortTermAdj/1
  LoadParaService_IncarReqShortTermAdj/1 -> Terminator_413/1
  LoadParaService_ReTXVReqRetCtrltoECU/1 -> Terminator_414/1
  Ground_54/1 -> LoadParaService_ReTXVReqShortTermAdj/1
  LoadParaService_ReTXVReqShortTermAdj/1 -> Terminator_415/1
  LoadParaService_ReadIncarMotorStatus/1 -> Terminator_416/1
  LoadParaService_ReadIncarMotorStatus/2 -> Terminator_417/1
  LoadParaService_ReadReTXVStatus/1 -> Terminator_418/1
  LoadParaService_ReadReTXVStatus/2 -> Terminator_419/1
  LoadParaService_SetBlwFbTriggerFlg/1 -> Terminator_420/1
  LoadParaService_VlclPwmRetCtrltoECU/1 -> Terminator_421/1
  Ground_55/1 -> LoadParaService_VlclPwmShortTermAdj/1
  LoadParaService_VlclPwmShortTermAdj/1 -> Terminator_422/1
  LoadParaService_VlclReadBlwDuty/1 -> Terminator_423/1
  LoadParaService_VlclReadBlwDuty/2 -> Terminator_424/1
  LoadParaService_VlclReadRearBlwDuty/1 -> Terminator_425/1
  LoadParaService_VlclReadRearBlwDuty/2 -> Terminator_426/1
  LoadParaService_VlclRearPwmRetCtrltoECU/1 -> Terminator_427/1
  Ground_56/1 -> LoadParaService_VlclRearPwmShortTermAdj/1
  LoadParaService_VlclRearPwmShortTermAdj/1 -> Terminator_428/1
  Ground_57/1 -> PwrParaService_GetMotorCailCnt/1
  PwrParaService_GetMotorCailCnt/1 -> Terminator_429/1
  PwrParaService_GetMotorCailCnt/2 -> Terminator_430/1
  Ground_58/1 -> PwrParaService_GetMotorCailNum/1
  PwrParaService_GetMotorCailNum/1 -> Terminator_431/1
  PwrParaService_GetMotorCailNum/2 -> Terminator_432/1
  Ground_59/1 -> PwrParaService_SetMotorCailCnt/1
  Ground_60/1 -> PwrParaService_SetMotorCailCnt/2
  PwrParaService_SetMotorCailCnt/1 -> Terminator_433/1
  Ground_61/1 -> PwrParaService_SetMotorCailNum/1
  Ground_62/1 -> PwrParaService_SetMotorCailNum/2
  PwrParaService_SetMotorCailNum/1 -> Terminator_434/1
  Ground_63/1 -> SensorParaService_GetValidValue/1
  SensorParaService_GetValidValue/1 -> Terminator_435/1
  SensorParaService_GetValidValue/2 -> Terminator_436/1
  Ground_64/1 -> SysServParaService_ReadCarConfigParaFaultCnt/1
  Ground_65/1 -> SysServParaService_ReadCarConfigParaFaultCnt/2
  SysServParaService_ReadCarConfigParaFaultCnt/1 -> Terminator_437/1
  SysServParaService_ReadCarConfigParaFaultCnt/2 -> Terminator_438/1
  SysServParaService_ReadData_DIDD005/1 -> Terminator_439/1
  SysServParaService_ReadData_DIDD005/2 -> Terminator_440/1
  SysServParaService_ReadData_DIDD09A/1 -> Terminator_441/1
  SysServParaService_ReadData_DIDD09A/2 -> Terminator_442/1
  SysServParaService_ReadData_DIDF190/1 -> Terminator_443/1
  SysServParaService_ReadData_DIDF190/2 -> Terminator_444/1
  SysServParaService_ReadData_DIDF1AB/1 -> Terminator_445/1
  SysServParaService_ReadData_DIDF1AB/2 -> Terminator_446/1
  Ground_66/1 -> SysServParaService_WriteData_DIDD09A/1
  SysServParaService_WriteData_DIDD09A/1 -> Terminator_447/1
  Ground_67/1 -> SysServParaService_WriteData_DIDF1AB/1
  Ground_68/1 -> SysServParaService_WriteData_DIDF1AB/2
  SysServParaService_WriteData_DIDF1AB/1 -> Terminator_448/1
  VgaCaliServive_SetAllCalibrate/1 -> Terminator_449/1
  VgaCaliServive_SetAllParaInit/1 -> Terminator_450/1
  Ground_69/1 -> VgaCaliServive_SetMotorCalibrate/1
  VgaCaliServive_SetMotorCalibrate/1 -> Terminator_451/1
  Ground_70/1 -> VgaCaliServive_SetMotorParaInit/1
  VgaCaliServive_SetMotorParaInit/1 -> Terminator_452/1
  Ground_71/1 -> VgaDtcService_GetElecErr/1
  VgaDtcService_GetElecErr/1 -> Terminator_453/1
  VgaDtcService_GetElecErr/2 -> Terminator_454/1
  Ground_72/1 -> VgaDtcService_GetRecErr/1
  VgaDtcService_GetRecErr/1 -> Terminator_455/1
  VgaDtcService_GetRecErr/2 -> Terminator_456/1
  Ground_73/1 -> VgaDtcService_GetStallErr/1
  VgaDtcService_GetStallErr/1 -> Terminator_457/1
  VgaDtcService_GetStallErr/2 -> Terminator_458/1
  Ground_74/1 -> VgaDtcService_GetTempErr/1
  VgaDtcService_GetTempErr/1 -> Terminator_459/1
  VgaDtcService_GetTempErr/2 -> Terminator_460/1
  Ground_75/1 -> VgaDtcService_GetVoltErr/1
  VgaDtcService_GetVoltErr/1 -> Terminator_461/1
  VgaDtcService_GetVoltErr/2 -> Terminator_462/1
  Ground_76/1 -> VgaIOCtrlService_FreezeMotorCurrentPosition/1
  VgaIOCtrlService_FreezeMotorCurrentPosition/1 -> Terminator_463/1
  Ground_77/1 -> VgaIOCtrlService_MotorReturnToECU/1
  VgaIOCtrlService_MotorReturnToECU/1 -> Terminator_464/1
  Ground_78/1 -> VgaIOCtrlService_MotorShortTermAdj/1
  Ground_79/1 -> VgaIOCtrlService_MotorShortTermAdj/2
  VgaIOCtrlService_MotorShortTermAdj/1 -> Terminator_465/1
  VgaParaService_GetAllMotorCaliStatus/1 -> Terminator_466/1
  VgaParaService_GetAllMotorCaliStatus/2 -> Terminator_467/1
  VgaParaService_GetCailRoutineStatus/1 -> Terminator_468/1
  VgaParaService_GetCailRoutineStatus/2 -> Terminator_469/1
  VgaParaService_GetFreshRecErrorStatus/1 -> Terminator_470/1
  VgaParaService_GetFreshRecErrorStatus/2 -> Terminator_471/1
  VgaParaService_GetFrntVgaErrorStatus/1 -> Terminator_472/1
  VgaParaService_GetFrntVgaErrorStatus/2 -> Terminator_473/1
  VgaParaService_GetMotorAllowSleepSts/1 -> Terminator_474/1
  VgaParaService_GetMotorAllowSleepSts/2 -> Terminator_475/1
  Ground_80/1 -> VgaParaService_GetMotorCaliStatus/1
  VgaParaService_GetMotorCaliStatus/1 -> Terminator_476/1
  VgaParaService_GetMotorCaliStatus/2 -> Terminator_477/1
  Ground_81/1 -> VgaParaService_GetMotorCurrentPosition/1
  VgaParaService_GetMotorCurrentPosition/1 -> Terminator_478/1
  VgaParaService_GetMotorCurrentPosition/2 -> Terminator_479/1
  Ground_82/1 -> VgaParaService_GetMotorCurrentPositionNew/1
  VgaParaService_GetMotorCurrentPositionNew/1 -> Terminator_480/1
  VgaParaService_GetMotorCurrentPositionNew/2 -> Terminator_481/1
  Ground_83/1 -> VgaParaService_GetMotorDidWorkingStatus/1
  VgaParaService_GetMotorDidWorkingStatus/1 -> Terminator_482/1
  VgaParaService_GetMotorDidWorkingStatus/2 -> Terminator_483/1
  Ground_84/1 -> VgaParaService_GetMotorMoveCnt/1
  VgaParaService_GetMotorMoveCnt/1 -> Terminator_484/1
  VgaParaService_GetMotorMoveCnt/2 -> Terminator_485/1
  Ground_85/1 -> VgaParaService_GetMotorReqPosition/1
  VgaParaService_GetMotorReqPosition/1 -> Terminator_486/1
  VgaParaService_GetMotorReqPosition/2 -> Terminator_487/1
  Ground_86/1 -> VgaParaService_GetMotorStepRange/1
  VgaParaService_GetMotorStepRange/1 -> Terminator_488/1
  VgaParaService_GetMotorStepRange/2 -> Terminator_489/1
  Ground_87/1 -> VgaParaService_GetMotorWorkingStatus/1
  VgaParaService_GetMotorWorkingStatus/1 -> Terminator_490/1
  VgaParaService_GetMotorWorkingStatus/2 -> Terminator_491/1
  VgaParaService_GetRearVgaErrorStatus/1 -> Terminator_492/1
  VgaParaService_GetRearVgaErrorStatus/2 -> Terminator_493/1
  Ground_88/1 -> VgaParaService_SetCailRoutineStatus/1
  VgaParaService_SetCailRoutineStatus/1 -> Terminator_494/1
  Ground_89/1 -> VgaParaService_SetMotorMoveCnt/1
  Ground_90/1 -> VgaParaService_SetMotorMoveCnt/2
  VgaParaService_SetMotorMoveCnt/1 -> Terminator_495/1
  Ground_91/1 -> VgaParaService_SetMotorRequestPosition/1
  Ground_92/1 -> VgaParaService_SetMotorRequestPosition/2
  VgaParaService_SetMotorRequestPosition/1 -> Terminator_496/1
  Ground_93/1 -> VgaParaService_SetMotorStepRange/1
  Ground_94/1 -> VgaParaService_SetMotorStepRange/2
  VgaParaService_SetMotorStepRange/1 -> Terminator_497/1
